simulink library "Renault_Library"  (Simulink 8.2)
settings: Solver=ode3  StartTime=0.0  StopTime=10.0  FixedStep=0.020  RelTol=1e-3
undefined symbols (not defined in the file or in its folder): ABC, AssystemProposal, b, chkval, CoeffA_C, CoeffB_C, CoeffMean_C, ConfTime_C, DiagDelay_C, DividePercent, FailureConfActive_C, FallingEdgeDuration_C, HighLevelThresholdVoltage_C, HighLevelVoltage_C, HighLevelVoltageTunable_C, InitValue_C, InputVoltRangeMax_C, InputVoltRangeMin_C, LowActiveContact_C, LowLevelThresholdVoltage_C, LowLevelVoltage_C, MaxOperatingVoltage_C, MinOperatingVoltage_C, mV2V_conversion, OUT1, OutOfRangeHigh_C, OutOfRangeLow_C, PinSupplySaturationMax, PinSupplySaturationMin, Res1Value_C, Res2Value_C, Res3Value_C, RisingEdgeDuration_C, RSA_CANSignalFailMonitoring, SampleTime_C, service14Table, service19Table, service22Table, service24Table, service28Table, service2ETable, service2FTable, service31Table, service87Table, SizeXStateDID2FTable, SizeXTable14, SizeXTable19, SizeXTable22, SizeXTable24, SizeXTable28, SizeXTable2E, SizeXTable2F, SizeXTable31, SizeXTable87, SizeYStateDID2FTable, SizeYTable14, SizeYTable19, SizeYTable22, SizeYTable24, SizeYTable28 ... (+22)
undefined symbols (not defined in the file): BatteryVoltage_ActivationThreshold_C, FrameStatus_AbsentValue_C, FrameStatus_ConfAbsentValue_C, MemThreshold_C, NAMEOFTHEMODEL_SAMPLE_TIME_CST, NbAbs1_C, NbConfAbs1_C, NbConfPres1_C, NbPres1_C, NOMDUSIGNAL_FaultConfTime_C, NOMDUSIGNAL_FaultRehabTime_C, NOMDUSIGNAL_switchvalue_C, NOMDUSIGNAL_unavailablevalue_C, PeriodFrame1_C, ResetTime_C, WakeUpTime_C
libraries: Renault_Library x112, simulink x75
(part 14 of 32)

== system Renault_Library/Services ECU/Mux/RSA_ConvertToMux ==
  DATA : Inport
      settings: AttributesFormatString=Type : %<OutDataTypeStr>
  Enable : EnablePort  StatesWhenEnabling=reset
  Compare : RelationalOperator  Operator===  OutDataTypeStr=boolean  InputSameDT=on
  Constant : Constant  DisableCoverage=on  Value=0  OutDataTypeStr=Inherit: Inherit via back propagation  SampleTime=-1  PreserveConstantTs=on
  Constant1 : Constant  Value=Offset  OutDataTypeStr=Inherit: Inherit via back propagation  SampleTime=-1
  Constant2 : Constant  Value=Divisor  OutDataTypeStr=Inherit: Inherit via back propagation  SampleTime=-1
  Constant3 : Constant  Value=Factor  OutDataTypeStr=Inherit: Inherit via back propagation  SampleTime=-1
  Constant4 : Constant  OutDataTypeStr=Inherit: Inherit via back propagation  SampleTime=-1
  Divide : Product  Inputs=*/  OutDataTypeStr=Inherit: Inherit via back propagation  RndMeth=Zero  InputSameDT=on
      settings: AttributesFormatString=Saturation:%<SaturateOnIntegerOverflow>
  Product : Product  OutDataTypeStr=Inherit: Inherit via back propagation  RndMeth=Zero  InputSameDT=on
      settings: AttributesFormatString=Saturation:%<SaturateOnIntegerOverflow>
  Sum : Sum  Inputs=+-  OutDataTypeStr=Inherit: Inherit via back propagation  RndMeth=Zero  InputSameDT=on
      settings: AttributesFormatString=Saturation:%<SaturateOnIntegerOverflow>
  Switch : Switch  Criteria=u2 ~= 0  RndMeth=Zero  InputSameDT=on
      settings: AttributesFormatString=Threshold : %<Threshold>\nSaturation:%<SaturateOnIntegerOverflow>
  SIGNAL : Outport  OutputWhenDisabled=reset  InitialOutput=0
      settings: AttributesFormatString=Type : %<OutDataTypeStr>\nInit :%<InitialOutput>
  wires:
  Constant/1 -> Compare/2
  Switch/1 -> Divide/2
  Constant4/1 -> Switch/1
  Compare/1 -> Switch/2
  Divide/1 -> SIGNAL/1
  Constant2/1 -> Compare/1, Switch/3
  Sum/1 -> Divide/1
  Constant1/1 -> Sum/2
  Product/1 -> Sum/1
  Constant3/1 -> Product/2
  DATA/1 -> Product/1

== system Renault_Library/Services ECU/Mux/RSA_FrameMonitoring ==
  FRAME_RECEIVED : Inport
      settings: AttributesFormatString=Type : %<OutDataTypeStr>
  Constant : Constant  Value=INCREMENT_COUNTER  OutDataTypeStr=Inherit: Inherit via back propagation  SampleTime=-1
  Constant1 : Constant  Value=MAX_COUNTER  OutDataTypeStr=Inherit: Inherit via back propagation  SampleTime=-1
  Constant2 : Constant  Value=MIN_COUNTER  OutDataTypeStr=Inherit: Inherit via back propagation  SampleTime=-1
  RSA_DetectChange : Renault_Library/Complex blocks/Logic & Bit Operations/RSA_DetectChange  Tag=[LIB]  vinit=0
  RelationalOperator : RelationalOperator  Operator===  OutDataTypeStr=boolean  InputSameDT=on
  Sum : Sum  OutDataTypeStr=Inherit: Inherit via back propagation  RndMeth=Zero  InputSameDT=on
      settings: AttributesFormatString=Saturation:%<SaturateOnIntegerOverflow>
  Switch : Switch  Criteria=u2 ~= 0  RndMeth=Zero  InputSameDT=on
      settings: AttributesFormatString=Threshold : %<Threshold>\nSaturation:%<SaturateOnIntegerOverflow>\nCriteria:%<Criteria>
  Switch1 : Switch  Criteria=u2 ~= 0  RndMeth=Zero  InputSameDT=on
      settings: AttributesFormatString=Threshold : %<Threshold>\nSaturation:%<SaturateOnIntegerOverflow>\nCriteria:%<Criteria>
  UnitDelay : UnitDelay  SampleTime=-1
      settings: AttributesFormatString=Initial value : %<X0>
  UnitDelay1 : UnitDelay  SampleTime=-1
      settings: AttributesFormatString=Initial value : %<X0>
  FRAME_SEND : Outport  OutputWhenDisabled=reset  InitialOutput=0
      settings: AttributesFormatString=Type : %<OutDataTypeStr>\nInit :%<InitialOutput>
  wires:
  Switch/1 -> FRAME_SEND/1, UnitDelay/1, UnitDelay1/1
  Constant/1 -> Sum/2
  RSA_DetectChange/1 -> Switch/2
  FRAME_RECEIVED/1 -> RSA_DetectChange/1
  UnitDelay/1 -> Switch/3
  UnitDelay1/1 -> Sum/1
  Sum/1 -> RelationalOperator/1, Switch1/3
  Constant1/1 -> RelationalOperator/2
  Constant2/1 -> Switch1/1
  RelationalOperator/1 -> Switch1/2
  Switch1/1 -> Switch/1

== system Renault_Library/Services ECU/Mux/RSA_FrameStatusMgt ==
  note: Frame_Update is equal to 1
      | when a frame has been received
      | (=1 each time the RX counter is incremented)
  note: Frame_Status:
      | = 1 for normally absent (absent but not expected) and init
      | = 2 for present
      | = 3 for anormally absent (absent and expected)
      | = 4 for anormally absent confirmed (absent and expected confirmed)
  note: A delay of NbAbs*Period*TimeOut is waited to declare an absent frame
      | A delay of NbConfAbs*Period*TimeOut is waited to confirm an absent frame
      | If NbConfPres = 0, there is no delay waited to rehabilite the frame
      | If NbPres = 0, stateflow is continuously waiting (without going back to ConfirmedAbsente State)
      | a NbConfPres Time to rehabilite the frame
      |
      | NbAbs, NbConf, Period and SampleTime are configurable parameters
  note: Fault_Management_Allowed = 1 when ECU is ON
      | Or when GADE is enabled
  Fault_Management_Allowed : Inport
  Frame_Update : Inport  Port=2
  DataTypeConversion : DataTypeConversion  RndMeth=Zero
      settings: AttributesFormatString=Saturation:%<SaturateOnIntegerOverflow>
  DataTypeConversion1 : DataTypeConversion  RndMeth=Zero
      settings: AttributesFormatString=Saturation:%<SaturateOnIntegerOverflow>
  FrameMonitoring : Stateflow chart  TreatAsAtomicUnit=on
  NbAbsCalib : Constant  Value=NbAbsCalib
  NbConfCalib : Constant  Value=NbConfCalib
  NbConfPresCalib : Constant  Value=NbConfPresCalib
  NbPresCalib : Constant  Value=NbPresCalib
  PeriodCalib : Constant  Value=PeriodCalib
  SampleTimeCalib : Constant  Value=SampleTimeCalib
  Terminator : Terminator
  Terminator1 : Terminator
  Frame_Status : Outport  InitialOutput=1
  wires:
  NbConfPresCalib/1 -> FrameMonitoring/8(NbConfPres)
  NbPresCalib/1 -> FrameMonitoring/7(NbPres)
  Fault_Management_Allowed/1 -> DataTypeConversion/1
  Frame_Update/1 -> DataTypeConversion1/1
  FrameMonitoring/1(Frame_Status) -> Frame_Status/1
  NbAbsCalib/1 -> FrameMonitoring/5(NbAbs)
  NbConfCalib/1 -> FrameMonitoring/6(NbConfAbs)
  PeriodCalib/1 -> FrameMonitoring/4(Period)
  FrameMonitoring/2(Cpt) -> Terminator/1
  FrameMonitoring/3(Cpt2) -> Terminator1/1
  SampleTimeCalib/1 -> FrameMonitoring/3(Ts)
  DataTypeConversion/1 -> FrameMonitoring/1(Fault_Management_Allowed)
  DataTypeConversion1/1 -> FrameMonitoring/2(Frame_Update)

== system Renault_Library/Services ECU/Mux/RSA_FrameUpdate ==
  note: Frame_Update is equal to 1
      | when a frame has been received
      | (=1 each time the RX counter is incremented)
  Frame_RX : Inport
  Fault_Management_Allowed : Inport  Port=2
  FrameUpdateAlwaysRunning : Constant  Value=FrameUpdateAlwaysRunning  OutDataTypeStr=Inherit: Inherit via back propagation  SampleTime=-1
  LogicalOperator1 : Logic  OutDataTypeStr=boolean
  RSA_DetectChange1 : Renault_Library/Complex blocks/Logic & Bit Operations/RSA_DetectChange  Tag=[LIB]  vinit=0
  Switch : Switch  Criteria=u2 ~= 0  RndMeth=Zero  InputSameDT=on
      settings: AttributesFormatString=Threshold : %<Threshold>\nSaturation:%<SaturateOnIntegerOverflow>\nCriteria:%<Criteria>
  Frame_Update : Outport  InitialOutput=0
  wires:
  Frame_RX/1 -> RSA_DetectChange1/1
  RSA_DetectChange1/1 -> LogicalOperator1/1, Switch/1
  Fault_Management_Allowed/1 -> LogicalOperator1/2
  Switch/1 -> Frame_Update/1
  FrameUpdateAlwaysRunning/1 -> Switch/2
  LogicalOperator1/1 -> Switch/3

== system Renault_Library/Services ECU/Mux/RSA_MuxMonitoring ==
  FRAME_RECEIVED : Inport  OutDataTypeStr=uint32
      settings: AttributesFormatString=Type : %<OutDataTypeStr>
  Enable : EnablePort
  CanFramePresence : Stateflow chart  TreatAsAtomicUnit=on
  FRAME_STATUS : Outport  OutDataTypeStr=uint32  OutputWhenDisabled=reset  InitialOutput=0
      settings: AttributesFormatString=Type : %<OutDataTypeStr>\nInit :%<InitialOutput>
  FRAME_PRESENT : Outport  Port=2  OutDataTypeStr=boolean  InitialOutput=0
      settings: AttributesFormatString=Type : %<OutDataTypeStr>\nInit :%<InitialOutput>
  wires:
  CanFramePresence/2(FRAME_PRESENT) -> FRAME_PRESENT/1
  CanFramePresence/1(FRAME_STATUS) -> FRAME_STATUS/1
  FRAME_RECEIVED/1 -> CanFramePresence/1(FRAME_RECEIVED)

== system Renault_Library/Services ECU/Mux/RSA_MuxSendFrame ==
  EVENT_DATA : Inport  OutDataTypeStr=boolean
      settings: AttributesFormatString=Type : %<OutDataTypeStr>
  Enable : EnablePort  StatesWhenEnabling=reset
  RSA_Send_Frame_FCT : Stateflow chart  TreatAsAtomicUnit=on
  FRAME_SEND : Outport  OutDataTypeStr=uint32  OutputWhenDisabled=reset  InitialOutput=0
      settings: AttributesFormatString=Type : %<OutDataTypeStr>\nInit :%<InitialOutput>
  FRAME_PRESENT : Outport  Port=2  OutDataTypeStr=boolean  OutputWhenDisabled=reset  InitialOutput=false
      settings: AttributesFormatString=Type : %<OutDataTypeStr>\nInit :%<InitialOutput>
  wires:
  RSA_Send_Frame_FCT/2(FRAME_PRESENT) -> FRAME_PRESENT/1
  EVENT_DATA/1 -> RSA_Send_Frame_FCT/1(EVENT_DATA)
  RSA_Send_Frame_FCT/1(CNT_FRAME_UPDATED) -> FRAME_SEND/1

== system Renault_Library/Services ECU/PowerSupply/RSA_PowerSupplyMgt ==
  note: Apc = PowerSupplyON = ECU activation switch
  note: EcuStatus= 0  --> Ecu off
      | EcuStatus= 1 --> Ecu on
      | EcuStatus= 2 --> Ecu Waking Up (Init)
      | EcuStatus= 3 --> Ecu Shutting Down (Reset)
  note: Read during Init or first step Ecu On when Init = 0
      | Write during Reset or last step Ecu On when Reset = 0
  note: ActivateEcu = 1 when BatteryVoltage > BattVoltageThreshold AND Apc is ON
      | ActivateEcu = 0 when Ecu is Off (EcuStatus = 0)
  note: WARNING: The writng operation is corresponding to powerlatch saving phase.
      | This need to be adapted for other specific writing sequence
  note: VoltageSupplyCutThreshold is a parameter to allow memorization when voltage is above its value.
      | If the voltage immediatly go under this threshold, there is no writing phase so no memorization.
      |
      | BattVoltageThreshold is a parameter that allow the activation of ECU.
      | If there is no voltage input, put the threshold to low value.
  BatteryVoltage : Inport
  Apc : Inport  Port=2
  BattVoltageThreshold : Constant  Value=BattVoltageThresholdCalib
  PowerSupMgt_Chart : Stateflow chart  TreatAsAtomicUnit=on
  ResetTimeCalib : Constant  Value=ResetTimeCalib
  SampleTimeCalib : Constant  Value=SampleTimeCalib
  VoltageSupplyCutThresholdCalib : Constant  Value=VoltageSupplyCutThresholdCalib
  WakeUpTimeCalib : Constant  Value=WakeUpTimeCalib
  ActivateEcu : Outport
  EcuStatus : Outport  Port=2
  Read : Outport  Port=3
  Write : Outport  Port=4
  wires:
  BattVoltageThreshold/1 -> PowerSupMgt_Chart/3(BatteryVoltageThreshold)
  PowerSupMgt_Chart/1(ActivateEcu) -> ActivateEcu/1
  PowerSupMgt_Chart/4(write) -> Write/1
  Apc/1 -> PowerSupMgt_Chart/1(Apc)
  PowerSupMgt_Chart/3(read) -> Read/1
  BatteryVoltage/1 -> PowerSupMgt_Chart/2(BatteryVoltage)
  PowerSupMgt_Chart/2(EcuStatus) -> EcuStatus/1
  WakeUpTimeCalib/1 -> PowerSupMgt_Chart/5(WakeUpTime)
  ResetTimeCalib/1 -> PowerSupMgt_Chart/6(ResetTime)
  VoltageSupplyCutThresholdCalib/1 -> PowerSupMgt_Chart/4(VoltageSupplyCutThreshold)
  SampleTimeCalib/1 -> PowerSupMgt_Chart/7(SampleTime)

== system Renault_Library/Standard blocks/Commonly Used Blocks/Subsystem ==
  In1 : Inport
  Out1 : Outport
  wires:
  In1/1 -> Out1/1

== system Renault_Library/Standard blocks/Discontinuities/SaturationDynamic ==
  up : Inport
      settings: AttributesFormatString=Type : %<OutDataTypeStr>
  u : Inport  Port=2
      settings: AttributesFormatString=Type : %<OutDataTypeStr>
  lo : Inport  Port=3
      settings: AttributesFormatString=Type : %<OutDataTypeStr>
  LowerRelop1 : RelationalOperator  DisableCoverage=on  Operator=>  OutDataTypeStr=boolean  InputSameDT=on
  Switch : Switch  Criteria=u2 ~= 0  OutDataTypeStr=Inherit: Inherit via back propagation  RndMeth=Zero  ZeroCross=off  InputSameDT=on
  Switch2 : Switch  Criteria=u2 ~= 0  OutMin=OutMin  OutMax=OutMax  OutDataTypeStr=Inherit: Inherit via back propagation  RndMeth=Zero  ZeroCross=off  InputSameDT=on
  UpperRelop : RelationalOperator  DisableCoverage=on  Operator=<  OutDataTypeStr=boolean  InputSameDT=on
  y : Outport
      settings: AttributesFormatString=Type : %<OutDataTypeStr>\nInit :%<InitialOutput>
  wires:
  [up] -> Switch2/1
  [u] -> UpperRelop/1
  [u] -> Switch/3
  [lo] -> Switch/1
  up/1 -> [up], LowerRelop1/2
  u/1 -> LowerRelop1/1, [u]
  lo/1 -> [lo], UpperRelop/2
  Switch/1 -> Switch2/3
  Switch2/1 -> y/1
  LowerRelop1/1 -> Switch2/2
  UpperRelop/1 -> Switch/2

== system Renault_Library/Standard blocks/Logic & Bit Operations/Extract Bits ==
  u : Inport
  DTProp1 : simulink/Signal Attributes/Data Type Propagation  PropDataType=fixdt(1, 16)  IfRefDouble=double  IfRefSingle=single  IsSigned=IsSigned1  NumBitsBase=NumBits1  NumBitsMult=0.5  NumBitsAdd=0  NumBitsAllowFinal=1:128  PropScaling=2^-10  ValuesUsedBestPrec=[5 -7]  SlopeBase=PosRange1  SlopeMult=2^0  SlopeAdd=0  BiasBase=Bias1  BiasMult=1  BiasAdd=0
  DTProp2 : simulink/Signal Attributes/Data Type Propagation  PropDataType=fixdt(1, 16)  IfRefDouble=double  IfRefSingle=single  IsSigned=IsSigned1  NumBitsBase=NumBits1  NumBitsMult=1  NumBitsAdd=0  NumBitsAllowFinal=1:128  PropScaling=1  ValuesUsedBestPrec=[5 -7]  SlopeBase=Slope1  SlopeMult=1  SlopeAdd=0  BiasBase=Bias1  BiasMult=1  BiasAdd=0
  Extract Desired Bits : DataTypeConversion  DisableCoverage=on
  Modify Scaling Only : DataTypeConversion  ConvertRealWorld=Stored Integer (SI)
  y : Outport
  wires:
  Modify Scaling Only/1 -> DTProp2/3, y/1
  u/1 -> Extract Desired Bits/1, DTProp1/2, DTProp1/1
  Extract Desired Bits/1 -> DTProp2/1, DTProp2/2, Modify Scaling Only/1, DTProp1/3

== system Renault_Library/Standard blocks/Model Wide Utilities/DocBlock ==
  EmptySubsystem : Subsystem

== system Renault_Library/Standard blocks/Model Wide Utilities/ModelInfo ==
  EmptySubsystem : Subsystem

== system Renault_Library/Standard blocks/Ports & Subsystems/AtomicSubsystem ==
  In : Inport
  Out : Outport
  wires:
  In/1 -> Out/1

== system Renault_Library/Standard blocks/Ports & Subsystems/CodeReuseSubsystem ==
  In1 : Inport
  Out1 : Outport
  wires:
  In1/1 -> Out1/1

== system Renault_Library/Standard blocks/Ports & Subsystems/EnabledSubsystem ==
  In1 : Inport
  Enable : EnablePort
      settings: AttributesFormatString=State : %<StatesWhenEnabling>
  Out1 : Outport
  wires:
  In1/1 -> Out1/1

== system Renault_Library/Standard blocks/Ports & Subsystems/EnabledandTriggeredSubsystem ==
  In1 : Inport
  Enable : EnablePort
  Trigger : TriggerPort
  Out1 : Outport
  wires:
  In1/1 -> Out1/1

== system Renault_Library/Standard blocks/Ports & Subsystems/ForIteratorSubsystem ==
  In1 : Inport
  ForIterator : ForIterator
  Out1 : Outport
  wires:
  In1/1 -> Out1/1

== system Renault_Library/Standard blocks/Ports & Subsystems/FunctionCallSubsystem ==
  In1 : Inport
  function_call : TriggerPort  TriggerType=function-call
  Out1 : Outport
  wires:
  In1/1 -> Out1/1

== system Renault_Library/Standard blocks/Ports & Subsystems/IfActionSubsystem ==
  In1 : Inport
  ActionPort : ActionPort
      settings: AttributesFormatString=State : %<InitializeStates>
  Out1 : Outport
  wires:
  In1/1 -> Out1/1

== system Renault_Library/Standard blocks/Ports & Subsystems/Subsystem ==
  In1 : Inport
  Out1 : Outport
  wires:
  In1/1 -> Out1/1

== system Renault_Library/Standard blocks/Ports & Subsystems/SwitchCaseActionSubsystem ==
  In1 : Inport
  ActionPort : ActionPort
  Out1 : Outport
  wires:
  In1/1 -> Out1/1

== system Renault_Library/Standard blocks/Ports & Subsystems/SwitchCaseActionSubsystem1 ==
  In1 : Inport
  ActionPort : ActionPort
  Out1 : Outport
  wires:
  In1/1 -> Out1/1

== system Renault_Library/Standard blocks/Ports & Subsystems/TriggeredSubsystem ==
  In1 : Inport
  Trigger : TriggerPort
      settings: AttributesFormatString=State : %<StatesWhenEnabling>
  Out1 : Outport
  wires:
  In1/1 -> Out1/1

== system Renault_Library/Standard blocks/Ports & Subsystems/Variant_Subsystem ==
  note: 1) Only subsystems can be added as variant choices at this level
      | 2) Blocks cannot be connected at this level as connectivity is
      | automatically determined at simulation, based on the active variant
  In : Inport
  Out : Outport

== system Renault_Library/Standard blocks/Ports & Subsystems/WhileIteratorSubsystem ==
  IC : Inport
  In1 : Inport  Port=2
  WhileIterator : WhileIterator
  Out1 : Outport
  wires:
  In1/1 -> Out1/1
  IC/1 -> WhileIterator/2

== system Renault_Library/Complex blocks/Discontinuities/RSA_Hysteresis_WithConf_V01_00/TurnOnDelay1 ==
  Delaytime : Inport  Tag=AM,In,0,1,Name,C;CMP,Port,PortWidth,SampleTime;
      settings: AttributesFormatString=Type : %<OutDataTypeStr>
  Signal : Inport  Tag=AM,In,0,1,Name,C;CMP,Port,PortWidth,SampleTime;  Port=2
      settings: AttributesFormatString=Type : %<OutDataTypeStr>
  Timestep1 : Inport  Tag=AM,In,0,1,Name,C;CMP,Port,PortWidth,SampleTime;  Port=3
      settings: AttributesFormatString=Type : %<OutDataTypeStr>
  Constant1 : Constant  Tag=AM,Constant,0,1,Value;CMP,Value;  Value=TIM1INI  OutDataTypeStr=Inherit: Inherit via back propagation
  Constant2 : Constant  Value=CONS_ONE  OutDataTypeStr=Inherit: Inherit via back propagation
  Constant3 : Constant  Value=TIM1INI  OutDataTypeStr=Inherit: Inherit via back propagation
  Constant4 : Constant  Value=CONS_ONE  OutDataTypeStr=Inherit: Inherit via back propagation
  Constant6 : Constant  Value=CONS_ZERO  OutDataTypeStr=Inherit: Inherit via back propagation
  Constant7 : Constant  Value=BOOSTING_FLP  OutDataTypeStr=uint32
  Constant8 : Constant  Value=BOOSTING_FXP  OutDataTypeStr=Inherit: Inherit via back propagation
  DataTypeConversion2 : DataTypeConversion  RndMeth=Zero
  DelayInput1 : UnitDelay  Description=\nStore in Global RAM  InitialCondition=timeoutinit  SampleTime=-1
      settings: AttributesFormatString=IC = %<X0>
  DelayInput2 : UnitDelay  Description=\nStore in Global RAM  InitialCondition=TIM1INI  SampleTime=-1
      settings: AttributesFormatString=IC = %<X0>
  EdgeRising : Subsystem  mask(EdgeRising){vinit=initinit}
      mask prompts: vinit "Initialisation"
      mask help: When a rising edge is used as input, the ouput is equal to one during the fall of the signal. Otherwise the output is zero.
      mask Display:
      | plot([0.2 0.4 0.4 0.6],[0.6 0.6 0.9 0.9]);
      | plot([0.2 0.4 0.4 0.4 0.6],[0.1 0.1 0.4 0.1 0.1])
  LogicalOperator : Logic  Tag=AM,Std,Inputs,1;CMP,Operator,Inputs;  OutDataTypeStr=boolean
  LogicalOperator1 : Logic  OutDataTypeStr=boolean
  Mux : Mux  Inputs=2
  Product : Product  OutDataTypeStr=Inherit: Inherit via back propagation  SaturateOnIntegerOverflow=on  RndMeth=Zero
  Product1 : Product  OutDataTypeStr=Inherit: Inherit via back propagation  SaturateOnIntegerOverflow=on  RndMeth=Zero
  Product2 : Product  OutDataTypeStr=uint32  SaturateOnIntegerOverflow=on  RndMeth=Zero
  RelationalOperator1 : RelationalOperator  Operator=>  OutDataTypeStr=boolean  ZeroCross=off  InputSameDT=on
  RelationalOperator2 : RelationalOperator  Operator=<=  OutDataTypeStr=boolean  ZeroCross=off  InputSameDT=on
  RelationalOperator3 : RelationalOperator  Operator=>  OutDataTypeStr=boolean  ZeroCross=off  InputSameDT=on
  Subtract4 : Sum  Inputs=-+  OutDataTypeStr=Inherit: Inherit via back propagation  RndMeth=Zero  InputSameDT=on
      settings: AttributesFormatString=SAT = %<SaturateOnIntegerOverflow>
  Sum : Sum  Inputs=+-  OutDataTypeStr=Inherit: Inherit via back propagation  RndMeth=Zero  InputSameDT=on
      settings: AttributesFormatString=SAT = %<SaturateOnIntegerOverflow>
  Switch{2..6} (5 alike) : Switch  Tag=GEN,Threshold;AM,Switch,2,1;CMP,Threshold;  Criteria=u2 ~= 0  RndMeth=Zero  SaturateOnIntegerOverflow=on  InputSameDT=on
      settings: AttributesFormatString=Criteria=%<Criteria>
  Terminator : Terminator
  Terminator1 : Terminator
  Terminator2 : Terminator
  Out : Outport  Tag=AM,Out,1,0,Name,NC;CMP,Port,OutputWhenDisabled,InitialOutput;
      settings: AttributesFormatString=Type : %<OutDataTypeStr>\nInit :%<InitialOutput>
  wires:
  Product/1 -> [Timestep1]
  Constant1/1 -> Switch5/3
  Switch5/1 -> Switch3/3
  RelationalOperator2/1 -> Switch5/2
  [EdgeRising] -> Switch3/2
  LogicalOperator1/1 -> LogicalOperator/2  "Timeout"
  Timestep1/1 -> Mux/1, Product/1, [Timestep]
  DelayInput1/1 -> Switch2/3
  Switch4/1 -> DelayInput2/1
  RelationalOperator1/1 -> Switch4/2, LogicalOperator1/2
  DelayInput2/1 -> RelationalOperator2/2, Sum/1
  Switch2/1 -> LogicalOperator1/1, DelayInput1/1
  Switch3/1 -> RelationalOperator1/2, Switch4/3
  EdgeRising/1(Out) -> [EdgeRising], Switch2/1, Switch2/2
  Signal/1 -> EdgeRising/1(In), LogicalOperator/1
  [Timestep1] -> Sum/2, RelationalOperator2/1
  Constant3/1 -> Switch4/1
  LogicalOperator/1 -> Out/1
  Mux/1 -> Terminator/1
  [Timestep] -> Product2/2, Subtract4/2
  Subtract4/1 -> RelationalOperator3/1
  [Timestep1] -> RelationalOperator1/1
  Constant6/1 -> RelationalOperator3/2
  Constant8/1 -> Switch6/3
  Constant7/1 -> Switch6/1
  Sum/1 -> Switch5/1
  Switch6/1 -> [tolcons]
  DataTypeConversion2/1 -> Subtract4/1
  Product2/1 -> DataTypeConversion2/1
  [CONS_ONE] -> Product1/2
  RelationalOperator3/1 -> Switch6/2
  Product1/1 -> Switch3/1
  Delaytime/1 -> Mux/2, Product1/1
  [CONS_ONE] -> Product/2
  Constant2/1 -> Product2/1
  [tolcons] -> Terminator1/1
  [CONS_ONE] -> Terminator2/1
  Constant4/1 -> [CONS_ONE]

== system Renault_Library/Complex blocks/Discontinuities/RSA_Hysteresis_WithConf_V01_00/TurnOnDelay2 ==
  Delaytime : Inport  Tag=AM,In,0,1,Name,C;CMP,Port,PortWidth,SampleTime;
      settings: AttributesFormatString=Type : %<OutDataTypeStr>
  Signal : Inport  Tag=AM,In,0,1,Name,C;CMP,Port,PortWidth,SampleTime;  Port=2
      settings: AttributesFormatString=Type : %<OutDataTypeStr>
  Timestep1 : Inport  Tag=AM,In,0,1,Name,C;CMP,Port,PortWidth,SampleTime;  Port=3
      settings: AttributesFormatString=Type : %<OutDataTypeStr>
  Constant1 : Constant  Tag=AM,Constant,0,1,Value;CMP,Value;  Value=TIM1INI  OutDataTypeStr=Inherit: Inherit via back propagation
  Constant2 : Constant  Value=CONS_ONE  OutDataTypeStr=Inherit: Inherit via back propagation
  Constant3 : Constant  Value=TIM1INI  OutDataTypeStr=Inherit: Inherit via back propagation
  Constant4 : Constant  Value=CONS_ONE  OutDataTypeStr=Inherit: Inherit via back propagation
  Constant6 : Constant  Value=CONS_ZERO  OutDataTypeStr=Inherit: Inherit via back propagation
  Constant7 : Constant  Value=BOOSTING_FLP  OutDataTypeStr=uint32
  Constant8 : Constant  Value=BOOSTING_FXP  OutDataTypeStr=Inherit: Inherit via back propagation
  DataTypeConversion2 : DataTypeConversion  RndMeth=Zero
  DelayInput1 : UnitDelay  Description=\nStore in Global RAM  InitialCondition=timeoutinit  SampleTime=-1
      settings: AttributesFormatString=IC = %<X0>
  DelayInput2 : UnitDelay  Description=\nStore in Global RAM  InitialCondition=TIM1INI  SampleTime=-1
      settings: AttributesFormatString=IC = %<X0>
  EdgeRising : Subsystem  mask(EdgeRising){vinit=initinit}
      mask prompts: vinit "Initialisation"
      mask help: When a rising edge is used as input, the ouput is equal to one during the fall of the signal. Otherwise the output is zero.
      mask Display:
      | plot([0.2 0.4 0.4 0.6],[0.6 0.6 0.9 0.9]);
      | plot([0.2 0.4 0.4 0.4 0.6],[0.1 0.1 0.4 0.1 0.1])
  LogicalOperator : Logic  Tag=AM,Std,Inputs,1;CMP,Operator,Inputs;  OutDataTypeStr=boolean
  LogicalOperator1 : Logic  OutDataTypeStr=boolean
  Mux : Mux  Inputs=2
  Product : Product  OutDataTypeStr=Inherit: Inherit via back propagation  SaturateOnIntegerOverflow=on  RndMeth=Zero
  Product1 : Product  OutDataTypeStr=Inherit: Inherit via back propagation  SaturateOnIntegerOverflow=on  RndMeth=Zero
  Product2 : Product  OutDataTypeStr=uint32  SaturateOnIntegerOverflow=on  RndMeth=Zero
  RelationalOperator1 : RelationalOperator  Operator=>  OutDataTypeStr=boolean  ZeroCross=off  InputSameDT=on
  RelationalOperator2 : RelationalOperator  Operator=<=  OutDataTypeStr=boolean  ZeroCross=off  InputSameDT=on
  RelationalOperator3 : RelationalOperator  Operator=>  OutDataTypeStr=boolean  ZeroCross=off  InputSameDT=on
  Subtract4 : Sum  Inputs=-+  OutDataTypeStr=Inherit: Inherit via back propagation  RndMeth=Zero  InputSameDT=on
      settings: AttributesFormatString=SAT = %<SaturateOnIntegerOverflow>
  Sum : Sum  Inputs=+-  OutDataTypeStr=Inherit: Inherit via back propagation  RndMeth=Zero  InputSameDT=on
      settings: AttributesFormatString=SAT = %<SaturateOnIntegerOverflow>
  Switch{2..6} (5 alike) : Switch  Tag=GEN,Threshold;AM,Switch,2,1;CMP,Threshold;  Criteria=u2 ~= 0  RndMeth=Zero  SaturateOnIntegerOverflow=on  InputSameDT=on
      settings: AttributesFormatString=Criteria=%<Criteria>
  Terminator : Terminator
  Terminator1 : Terminator
  Terminator2 : Terminator
  Out : Outport  Tag=AM,Out,1,0,Name,NC;CMP,Port,OutputWhenDisabled,InitialOutput;
      settings: AttributesFormatString=Type : %<OutDataTypeStr>\nInit :%<InitialOutput>
  wires:
  Product/1 -> [Timestep1]
  Constant1/1 -> Switch5/3
  Switch5/1 -> Switch3/3
  RelationalOperator2/1 -> Switch5/2
  [EdgeRising] -> Switch3/2
  LogicalOperator1/1 -> LogicalOperator/2  "Timeout"
  Timestep1/1 -> Mux/1, Product/1, [Timestep]
  DelayInput1/1 -> Switch2/3
  Switch4/1 -> DelayInput2/1
  RelationalOperator1/1 -> Switch4/2, LogicalOperator1/2
  DelayInput2/1 -> RelationalOperator2/2, Sum/1
  Switch2/1 -> LogicalOperator1/1, DelayInput1/1
  Switch3/1 -> RelationalOperator1/2, Switch4/3
  EdgeRising/1(Out) -> [EdgeRising], Switch2/1, Switch2/2
  Signal/1 -> EdgeRising/1(In), LogicalOperator/1
  [Timestep1] -> Sum/2, RelationalOperator2/1
  Constant3/1 -> Switch4/1
  LogicalOperator/1 -> Out/1
  Mux/1 -> Terminator/1
  [Timestep] -> Product2/2, Subtract4/2
  Subtract4/1 -> RelationalOperator3/1
  [Timestep1] -> RelationalOperator1/1
  Constant6/1 -> RelationalOperator3/2
  Constant8/1 -> Switch6/3
  Constant7/1 -> Switch6/1
  Sum/1 -> Switch5/1
  Switch6/1 -> [tolcons]
  DataTypeConversion2/1 -> Subtract4/1
  Product2/1 -> DataTypeConversion2/1
  [CONS_ONE] -> Product1/2
  RelationalOperator3/1 -> Switch6/2
  Product1/1 -> Switch3/1
  Delaytime/1 -> Mux/2, Product1/1
  [CONS_ONE] -> Product/2
  Constant2/1 -> Product2/1
  [tolcons] -> Terminator1/1
  [CONS_ONE] -> Terminator2/1
  Constant4/1 -> [CONS_ONE]

== system Renault_Library/Complex blocks/Lookup Tables/RSA_LookupTableDynamic_v02_01/Sat_blk ==
  Threshold_Upper : Inport
      settings: AttributesFormatString=Type : %<OutDataTypeStr>
  In : Inport  Port=2
      settings: AttributesFormatString=Type : %<OutDataTypeStr>
  Min : MinMax  Inputs=2  OutDataTypeStr=Inherit: Inherit via back propagation  RndMeth=Zero  SaturateOnIntegerOverflow=on  InputSameDT=on
  Out : Outport
      settings: AttributesFormatString=Type : %<OutDataTypeStr>\nInit :%<InitialOutput>
  wires:
  Threshold_Upper/1 -> [Threshold_Upper]
  In/1 -> Min/1
  Min/1 -> Out/1
  [Threshold_Upper] -> Min/2

== system Renault_Library/Complex blocks/Math Operations/RSA_DivisionByZero/Perform_Division ==
  num : Inport  Tag=AM,In,0,1,Name,C;CMP,Port,PortWidth,SampleTime;
      settings: AttributesFormatString=Type : %<OutDataTypeStr>
  den : Inport  Tag=AM,In,0,1,Name,C;CMP,Port,PortWidth,SampleTime;  Port=2
      settings: AttributesFormatString=Type : %<OutDataTypeStr>
  Enable_Division : EnablePort  Tag=GEN,StatesWhenEnabling;AM,In,0,1,Name,NC;CMP,StatesWhenEnabling;
      settings: AttributesFormatString=<states=%<statesWhenEnabling>>
  Divide : Product  Tag=[LIB]  Inputs=*/  OutDataTypeStr=Inherit: Inherit via back propagation  SaturateOnIntegerOverflow=on  RndMeth=Zero
      settings: AttributesFormatString=SAT = %<SaturateOnIntegerOverflow>
  ans : Outport  Tag=AM,Out,1,0,Name,NC;CMP,Port,OutputWhenDisabled,InitialOutput;  InitialOutput=0
      settings: AttributesFormatString=Init :%<InitialOutput>\nDisabled : %<OutputWhenDisabled>
  wires:
  Divide/1 -> ans/1
  den/1 -> Divide/2
  num/1 -> Divide/1

== system Renault_Library/Complex blocks/Signal Routing/RSA_SlopeSwitch2Inputs/Signal_Change_Compute ==
  Switch_Cmd : Inport
      settings: AttributesFormatString=Type : %<OutDataTypeStr>
  Relational_Operator : RelationalOperator  Operator=~=  OutDataTypeStr=boolean  InputSameDT=on
  UnitDelay : UnitDelay  InitialCondition=SS2ININI  SampleTime=-1
  Signal_Change : Outport
      settings: AttributesFormatString=Type : %<OutDataTypeStr>\nInit :%<InitialOutput>
  wires:
  Relational_Operator/1 -> Signal_Change/1
  Switch_Cmd/1 -> Relational_Operator/1, UnitDelay/1
  UnitDelay/1 -> Relational_Operator/2

== system Renault_Library/Complex blocks/Signal Routing/RSA_SlopeSwitch2Inputs/Slope_On_Block ==
  Signal_Change : Inport  Tag=AM,In,0,1,Name,C;CMP,Port,PortWidth,SampleTime;
      settings: AttributesFormatString=Type : %<OutDataTypeStr>
  Input : Inport  Tag=AM,In,0,1,Name,C;CMP,Port,PortWidth,SampleTime;  Port=2
      settings: AttributesFormatString=Type : %<OutDataTypeStr>
  Pos_Slope : Inport  Tag=AM,In,0,1,Name,C;CMP,Port,PortWidth,SampleTime;  Port=3
      settings: AttributesFormatString=Type : %<OutDataTypeStr>
  Neg_Slope : Inport  Tag=AM,In,0,1,Name,C;CMP,Port,PortWidth,SampleTime;  Port=4
      settings: AttributesFormatString=Type : %<OutDataTypeStr>
  Prev_Output : Inport  Port=5
      settings: AttributesFormatString=Type : %<OutDataTypeStr>
  Enable_Slope_On_Block : EnablePort  StatesWhenEnabling=reset
  Error_Due_To_High_Slope_Test : Subsystem
  Output_Comparision : Subsystem
  Output_Not_Equals_Input : Outport  Tag=AM,Out,1,0,Name,NC;CMP,Port,OutputWhenDisabled,InitialOutput;
      settings: AttributesFormatString=Init :%<InitialOutput>\nDisabled : %<OutputWhenDisabled>
  Output : Outport  Tag=AM,Out,1,0,Name,NC;CMP,Port,OutputWhenDisabled,InitialOutput;  Port=2
      settings: AttributesFormatString=Init :%<InitialOutput>\nDisabled : %<OutputWhenDisabled>
  wires:
  Output_Comparision/4(RelOp1_out) -> [RelOp1_out]
  Output_Comparision/3(RelOp2_out) -> [RelOp2_out]
  Neg_Slope/1 -> Output_Comparision/5(Neg_Slope)
  Input/1 -> Output_Comparision/2(Input)
  Pos_Slope/1 -> Output_Comparision/3(Pos_Slope)
  Prev_Output/1 -> Output_Comparision/4(Prev_Output)
  Error_Due_To_High_Slope_Test/1(Test_passed) -> Output_Comparision/1(Test_passed)
  Output_Comparision/2(Output) -> Output/1
  Output_Comparision/1(Output_Not_Equals_Input) -> Output_Not_Equals_Input/1
  [RelOp2_out] -> Error_Due_To_High_Slope_Test/3(RelOp2_out)
  [RelOp1_out] -> Error_Due_To_High_Slope_Test/2(RelOp1_out)
  Signal_Change/1 -> Error_Due_To_High_Slope_Test/1(Signal_Change)

== system Renault_Library/Complex blocks/Signal Routing/RSA_SlopeSwitch2Inputs/Slope_On_Compute ==
  Signal_Change : Inport  Tag=AM,In,0,1,Name,C;CMP,Port,PortWidth,SampleTime;
      settings: AttributesFormatString=Type : %<OutDataTypeStr>
  Output_Not_Equals_Input : Inport  Tag=AM,In,0,1,Name,C;CMP,Port,PortWidth,SampleTime;  Port=2
      settings: AttributesFormatString=Type : %<OutDataTypeStr>
  Constant1 : Constant  Value=false  OutDataTypeStr=Inherit: Inherit via back propagation  SampleTime=-1
  LogicalOperator : Logic  Operator=OR  OutDataTypeStr=boolean
  Switch1 : Switch  Tag=GEN,Threshold;AM,Switch,2,1;CMP,Threshold;  Criteria=u2 ~= 0  RndMeth=Zero  SaturateOnIntegerOverflow=on  InputSameDT=on
      settings: AttributesFormatString=Criteria=%<Criteria>
  UnitDelay3 : UnitDelay  InitialCondition=true  SampleTime=-1
  Slope_On : Outport  Tag=AM,Out,1,0,Name,NC;CMP,Port,OutputWhenDisabled,InitialOutput;
      settings: AttributesFormatString=Type : %<OutDataTypeStr>\nInit :%<InitialOutput>
  wires:
  Signal_Change/1 -> Switch1/3
  Constant1/1 -> UnitDelay3/1, Switch1/1
  Switch1/1 -> LogicalOperator/2
  UnitDelay3/1 -> Switch1/2
  LogicalOperator/1 -> Slope_On/1
  Output_Not_Equals_Input/1 -> LogicalOperator/1

== system Renault_Library/Complex blocks/Timers & Counters/RSA_Timer/EdgeRising ==
  In : Inport  Tag=AM,In,0,1,Name,C;CMP,Port,PortWidth,SampleTime;
      settings: AttributesFormatString=Type : %<OutDataTypeStr>
  DelayInput1 : UnitDelay  Description=\nStore in Global RAM  InitialCondition=vinit  SampleTime=-1
  LogicalOperator1 : Logic  OutDataTypeStr=boolean
  LogicalOperator3 : Logic  Operator=NOT  Inputs=1  OutDataTypeStr=boolean
  Out : Outport  Tag=AM,Out,1,0,Name,NC;CMP,Port,OutputWhenDisabled,InitialOutput;
      settings: AttributesFormatString=Type : %<OutDataTypeStr>\nInit :%<InitialOutput>
  wires:
  LogicalOperator1/1 -> Out/1
  In/1 -> LogicalOperator1/1, DelayInput1/1
  DelayInput1/1 -> LogicalOperator3/1
  LogicalOperator3/1 -> LogicalOperator1/2

== system Renault_Library/Complex blocks/Timers & Counters/RSA_Timer1/EdgeRising ==
  In : Inport  Tag=AM,In,0,1,Name,C;CMP,Port,PortWidth,SampleTime;
      settings: AttributesFormatString=Type : %<OutDataTypeStr>
  DelayInput1 : UnitDelay  Description=\nStore in Global RAM  InitialCondition=vinit  SampleTime=-1
  LogicalOperator1 : Logic  OutDataTypeStr=boolean
  LogicalOperator3 : Logic  Operator=NOT  Inputs=1  OutDataTypeStr=boolean
  Out : Outport  Tag=AM,Out,1,0,Name,NC;CMP,Port,OutputWhenDisabled,InitialOutput;
      settings: AttributesFormatString=Type : %<OutDataTypeStr>\nInit :%<InitialOutput>
  wires:
  LogicalOperator3/1 -> LogicalOperator1/2
  DelayInput1/1 -> LogicalOperator3/1
  In/1 -> DelayInput1/1, LogicalOperator1/1
  LogicalOperator1/1 -> Out/1

== system Renault_Library/Complex blocks/Timers & Counters/RSA_Timer1_v01_04/EdgeRising ==
  In : Inport  Tag=AM,In,0,1,Name,C;CMP,Port,PortWidth,SampleTime;
      settings: AttributesFormatString=Type : %<OutDataTypeStr>
  DelayInput1 : UnitDelay  Description=\nStore in Global RAM  InitialCondition=vinit  SampleTime=-1
  LogicalOperator1 : Logic  OutDataTypeStr=boolean
  LogicalOperator3 : Logic  Operator=NOT  Inputs=1  OutDataTypeStr=boolean
  Out : Outport  Tag=AM,Out,1,0,Name,NC;CMP,Port,OutputWhenDisabled,InitialOutput;
      settings: AttributesFormatString=Type : %<OutDataTypeStr>\nInit :%<InitialOutput>
  wires:
  LogicalOperator3/1 -> LogicalOperator1/2
  DelayInput1/1 -> LogicalOperator3/1
  In/1 -> DelayInput1/1, LogicalOperator1/1
  LogicalOperator1/1 -> Out/1

== system Renault_Library/Complex blocks/Timers & Counters/RSA_Timer1_v01_05/EdgeRising ==
  In : Inport  Tag=AM,In,0,1,Name,C;CMP,Port,PortWidth,SampleTime;
      settings: AttributesFormatString=Type : %<OutDataTypeStr>
  DelayInput1 : UnitDelay  Description=\nStore in Global RAM  InitialCondition=vinit  SampleTime=-1
      settings: AttributesFormatString=IC = %<X0>
  LogicalOperator1 : Logic  OutDataTypeStr=boolean
  LogicalOperator3 : Logic  Operator=NOT  Inputs=1  OutDataTypeStr=boolean
  Out : Outport  Tag=AM,Out,1,0,Name,NC;CMP,Port,OutputWhenDisabled,InitialOutput;
      settings: AttributesFormatString=Type : %<OutDataTypeStr>\nInit :%<InitialOutput>
  wires:
  LogicalOperator3/1 -> LogicalOperator1/2
  DelayInput1/1 -> LogicalOperator3/1
  In/1 -> DelayInput1/1, LogicalOperator1/1
  LogicalOperator1/1 -> Out/1

== system Renault_Library/Complex blocks/Timers & Counters/RSA_Timer1_v01_06/EdgeRising ==
  In : Inport  Tag=AM,In,0,1,Name,C;CMP,Port,PortWidth,SampleTime;
      settings: AttributesFormatString=Type : %<OutDataTypeStr>
  DelayInput1 : UnitDelay  Description=\nStore in Global RAM  InitialCondition=vinit  SampleTime=-1
      settings: AttributesFormatString=IC = %<X0>
  LogicalOperator1 : Logic  OutDataTypeStr=boolean
  LogicalOperator3 : Logic  Operator=NOT  Inputs=1  OutDataTypeStr=boolean
  Out : Outport  Tag=AM,Out,1,0,Name,NC;CMP,Port,OutputWhenDisabled,InitialOutput;
      settings: AttributesFormatString=Type : %<OutDataTypeStr>\nInit :%<InitialOutput>
  wires:
  LogicalOperator3/1 -> LogicalOperator1/2
  DelayInput1/1 -> LogicalOperator3/1
  In/1 -> DelayInput1/1, LogicalOperator1/1
  LogicalOperator1/1 -> Out/1

== system Renault_Library/Complex blocks/Timers & Counters/RSA_Timer_v01_04/EdgeRising ==
  In : Inport  Tag=AM,In,0,1,Name,C;CMP,Port,PortWidth,SampleTime;
      settings: AttributesFormatString=Type : %<OutDataTypeStr>
  DelayInput1 : UnitDelay  Description=\nStore in Global RAM  InitialCondition=vinit  SampleTime=-1
      settings: AttributesFormatString=IC = %<X0>
  LogicalOperator1 : Logic  OutDataTypeStr=boolean
  LogicalOperator3 : Logic  Operator=NOT  Inputs=1  OutDataTypeStr=boolean
  Out : Outport  Tag=AM,Out,1,0,Name,NC;CMP,Port,OutputWhenDisabled,InitialOutput;
      settings: AttributesFormatString=Type : %<OutDataTypeStr>\nInit :%<InitialOutput>
  wires:
  LogicalOperator3/1 -> LogicalOperator1/2
  DelayInput1/1 -> LogicalOperator3/1
  In/1 -> DelayInput1/1, LogicalOperator1/1
  LogicalOperator1/1 -> Out/1

== system Renault_Library/Complex blocks/Timers & Counters/RSA_Timer_v01_05/EdgeRising ==
  In : Inport  Tag=AM,In,0,1,Name,C;CMP,Port,PortWidth,SampleTime;
      settings: AttributesFormatString=Type : %<OutDataTypeStr>
  DelayInput1 : UnitDelay  Description=\nStore in Global RAM  InitialCondition=vinit  SampleTime=-1
      settings: AttributesFormatString=IC = %<X0>
  LogicalOperator1 : Logic  OutDataTypeStr=boolean
  LogicalOperator3 : Logic  Operator=NOT  Inputs=1  OutDataTypeStr=boolean
  Out : Outport  Tag=AM,Out,1,0,Name,NC;CMP,Port,OutputWhenDisabled,InitialOutput;
      settings: AttributesFormatString=Type : %<OutDataTypeStr>\nInit :%<InitialOutput>
  wires:
  LogicalOperator3/1 -> LogicalOperator1/2
  DelayInput1/1 -> LogicalOperator3/1
  In/1 -> DelayInput1/1, LogicalOperator1/1
  LogicalOperator1/1 -> Out/1

== system Renault_Library/Complex blocks/Timers & Counters/RSA_Timer_v01_05/RSA_CompareToZero ==
  u : Inport
      settings: AttributesFormatString=Type : %<OutDataTypeStr>
  Compare : RelationalOperator  Operator=~=  OutDataTypeStr=boolean  InputSameDT=on
  Constant : Constant  DisableCoverage=on  Value=cons_zero  OutDataTypeStr=Inherit: Inherit via back propagation  SampleTime=-1  PreserveConstantTs=on
  y : Outport
      settings: AttributesFormatString=Type : %<OutDataTypeStr>\nInit :%<InitialOutput>
  wires:
  Compare/1 -> y/1
  u/1 -> Compare/1
  Constant/1 -> Compare/2

== system Renault_Library/Complex blocks/Timers & Counters/RSA_Timer_v01_05/RSA_DataTypeDuplicate_v01_03 ==
  In1 : Inport
  In2 : Inport  Port=2
  Mux : Mux  Inputs=2
  Terminator : Terminator
  wires:
  Mux/1 -> Terminator/1
  In1/1 -> Mux/1
  In2/1 -> Mux/2

== system Renault_Library/Complex blocks/Timers & Counters/RSA_Timer_v01_06/EdgeRising ==
  In : Inport  Tag=AM,In,0,1,Name,C;CMP,Port,PortWidth,SampleTime;
      settings: AttributesFormatString=Type : %<OutDataTypeStr>
  DelayInput1 : UnitDelay  Description=\nStore in Global RAM  InitialCondition=vinit  SampleTime=-1
      settings: AttributesFormatString=IC = %<X0>
  LogicalOperator1 : Logic  OutDataTypeStr=boolean
  LogicalOperator3 : Logic  Operator=NOT  Inputs=1  OutDataTypeStr=boolean
  Out : Outport  Tag=AM,Out,1,0,Name,NC;CMP,Port,OutputWhenDisabled,InitialOutput;
      settings: AttributesFormatString=Type : %<OutDataTypeStr>\nInit :%<InitialOutput>
  wires:
  LogicalOperator3/1 -> LogicalOperator1/2
  DelayInput1/1 -> LogicalOperator3/1
  In/1 -> DelayInput1/1, LogicalOperator1/1
  LogicalOperator1/1 -> Out/1

== system Renault_Library/Complex blocks/Timers & Counters/RSA_Timer_v01_07/EdgeRising ==
  In : Inport  Tag=AM,In,0,1,Name,C;CMP,Port,PortWidth,SampleTime;
      settings: AttributesFormatString=Type : %<OutDataTypeStr>
  DelayInput1 : UnitDelay  Description=\nStore in Global RAM  InitialCondition=vinit  SampleTime=-1
      settings: AttributesFormatString=IC = %<X0>
  LogicalOperator1 : Logic  OutDataTypeStr=boolean
  LogicalOperator3 : Logic  Operator=NOT  Inputs=1  OutDataTypeStr=boolean
  Out : Outport  Tag=AM,Out,1,0,Name,NC;CMP,Port,OutputWhenDisabled,InitialOutput;
      settings: AttributesFormatString=Type : %<OutDataTypeStr>\nInit :%<InitialOutput>
  wires:
  LogicalOperator3/1 -> LogicalOperator1/2
  DelayInput1/1 -> LogicalOperator3/1
  In/1 -> DelayInput1/1, LogicalOperator1/1
  LogicalOperator1/1 -> Out/1

== system Renault_Library/Complex blocks/Timers & Counters/RSA_TurnOffDelay_v01_00/FallingEdge ==
  In : Inport  Tag=AM,In,0,1,Name,C;CMP,Port,PortWidth,SampleTime;
      settings: AttributesFormatString=Type : %<OutDataTypeStr>
  DelayInput1 : UnitDelay  Description=\nStore in Global RAM  InitialCondition=initinit  SampleTime=-1
      settings: AttributesFormatString=IC = %<X0>
  LogicalOperator1 : Logic  OutDataTypeStr=boolean
  LogicalOperator3 : Logic  Operator=NOT  Inputs=1  OutDataTypeStr=boolean
  Out : Outport  Tag=AM,Out,1,0,Name,NC;CMP,Port,OutputWhenDisabled,InitialOutput;
      settings: AttributesFormatString=Type : %<OutDataTypeStr>\nInit :%<InitialOutput>
  wires:
  DelayInput1/1 -> LogicalOperator1/2
  In/1 -> LogicalOperator3/1, DelayInput1/1
  LogicalOperator3/1 -> LogicalOperator1/1
  LogicalOperator1/1 -> Out/1

== system Renault_Library/Complex blocks/Timers & Counters/RSA_TurnOnDelay2_v01_04/RSA_CompareToZero ==
  u : Inport
      settings: AttributesFormatString=Type : %<OutDataTypeStr>
  Compare : RelationalOperator  Operator=~=  OutDataTypeStr=boolean  InputSameDT=on
  Constant : Constant  DisableCoverage=on  Value=cons_zero  OutDataTypeStr=Inherit: Inherit via back propagation  SampleTime=-1  PreserveConstantTs=on
  y : Outport
      settings: AttributesFormatString=Type : %<OutDataTypeStr>\nInit :%<InitialOutput>
  wires:
  Compare/1 -> y/1
  u/1 -> Compare/1
  Constant/1 -> Compare/2

== system Renault_Library/Complex blocks/Timers & Counters/RSA_TurnOnDelay2_v01_04/RSA_DataTypeDuplicate_v01_03 ==
  In1 : Inport
  In2 : Inport  Port=2
  Mux : Mux  Inputs=2
  Terminator : Terminator
  wires:
  Mux/1 -> Terminator/1
  In1/1 -> Mux/1
  In2/1 -> Mux/2

== system Renault_Library/Complex blocks/Timers & Counters/RSA_TurnOnDelay_v01_04/EdgeRising ==
  In : Inport  Tag=AM,In,0,1,Name,C;CMP,Port,PortWidth,SampleTime;
      settings: AttributesFormatString=Type : %<OutDataTypeStr>
  DelayInput1 : UnitDelay  Description=\nStore in Global RAM  InitialCondition=vinit  SampleTime=-1
  LogicalOperator1 : Logic  OutDataTypeStr=boolean
  LogicalOperator3 : Logic  Operator=NOT  Inputs=1  OutDataTypeStr=boolean
  Out : Outport  Tag=AM,Out,1,0,Name,NC;CMP,Port,OutputWhenDisabled,InitialOutput;
      settings: AttributesFormatString=Type : %<OutDataTypeStr>\nInit :%<InitialOutput>
  wires:
  LogicalOperator3/1 -> LogicalOperator1/2
  DelayInput1/1 -> LogicalOperator3/1
  In/1 -> DelayInput1/1, LogicalOperator1/1
  LogicalOperator1/1 -> Out/1

== system Renault_Library/Complex blocks/Timers & Counters/RSA_TurnOnDelay_v01_05/EdgeRising ==
  In : Inport  Tag=AM,In,0,1,Name,C;CMP,Port,PortWidth,SampleTime;
      settings: AttributesFormatString=Type : %<OutDataTypeStr>
  DelayInput1 : UnitDelay  Description=\nStore in Global RAM  InitialCondition=vinit  SampleTime=-1
      settings: AttributesFormatString=IC = %<X0>
  LogicalOperator1 : Logic  OutDataTypeStr=boolean
  LogicalOperator3 : Logic  Operator=NOT  Inputs=1  OutDataTypeStr=boolean
  Out : Outport  Tag=AM,Out,1,0,Name,NC;CMP,Port,OutputWhenDisabled,InitialOutput;
      settings: AttributesFormatString=Type : %<OutDataTypeStr>\nInit :%<InitialOutput>
  wires:
  LogicalOperator3/1 -> LogicalOperator1/2
  DelayInput1/1 -> LogicalOperator3/1
  In/1 -> DelayInput1/1, LogicalOperator1/1
  LogicalOperator1/1 -> Out/1

== system Renault_Library/Services ECU/Commonly_Used_Blocks/RSA_ServECU_EnaOutContact/ActiveStateAdaptation ==
  LogicalState : Inport
  DataTypeConversion2 : DataTypeConversion  RndMeth=Zero
  LogicalOperator2 : Logic  Operator=NOT  OutDataTypeStr=boolean
  RiseTime3 : Constant  Value=LowActiveContact_C  OutDataTypeStr=Inherit: Inherit via back propagation  SampleTime=-1
  Switch1 : Switch  Criteria=u2 ~= 0  RndMeth=Zero  InputSameDT=on
  AdaptedLogicalState : Outport  OutputWhenDisabled=reset  InitialOutput=0
  wires:
  Switch1/1 -> AdaptedLogicalState/1
  LogicalState/1 -> DataTypeConversion2/1
  LogicalOperator2/1 -> Switch1/1
  RiseTime3/1 -> Switch1/2
  DataTypeConversion2/1 -> Switch1/3, LogicalOperator2/1

== system Renault_Library/Services ECU/Commonly_Used_Blocks/RSA_ServECU_EnaOutContact/SwitchWithSlope ==
  Switch_Cmd : Inport
  RiseTime : Inport  Port=2
  FallTime : Inport  Port=3
  LowLevelVoltage : Inport  Port=4
  HighLevelVoltage : Inport  Port=5
  Enable : EnablePort  StatesWhenEnabling=reset
  Add1 : Sum  Inputs=-+  RndMeth=Zero  InputSameDT=on
  Add2 : Sum  Inputs=-+  RndMeth=Zero  InputSameDT=on
  RSA_ADivB1 : Renault_Library/Complex blocks/Math Operations/RSA_ADivB  Tag=[LIB]
  RSA_ADivB2 : Renault_Library/Complex blocks/Math Operations/RSA_ADivB  Tag=[LIB]
  RSA_SlopeSwitch2Inputs : Renault_Library/Complex blocks/Signal Routing/RSA_SlopeSwitch2Inputs  Tag=[LIB]  st=SampleTimeSec_C
  Out1 : Outport  OutputWhenDisabled=reset  InitialOutput=0
  Out2 : Outport  Port=2  OutputWhenDisabled=reset  InitialOutput=0
  wires:
  LowLevelVoltage/1 -> [LowLevelVoltage], RSA_SlopeSwitch2Inputs/4
  HighLevelVoltage/1 -> [HighLevelVoltage], RSA_SlopeSwitch2Inputs/5
  Switch_Cmd/1 -> RSA_SlopeSwitch2Inputs/1
  RSA_SlopeSwitch2Inputs/1 -> Out1/1
  RSA_SlopeSwitch2Inputs/2 -> Out2/1
  [HighLevelVoltage] -> Add2/1
  Add2/1 -> RSA_ADivB1/1
  [LowLevelVoltage] -> Add2/2
  Add1/1 -> RSA_ADivB2/1
  [HighLevelVoltage] -> Add1/2
  [LowLevelVoltage] -> Add1/1
  RiseTime/1 -> RSA_ADivB2/2
  RSA_ADivB2/1 -> RSA_SlopeSwitch2Inputs/2
  RSA_ADivB1/1 -> RSA_SlopeSwitch2Inputs/3
  FallTime/1 -> RSA_ADivB1/2

== system Renault_Library/Services ECU/Commonly_Used_Blocks/RSA_ServECU_EnaOutContact/SwitchWithoutSlope ==
  In1 : Inport
  Switch_Cmd : Inport  Port=2
  In0 : Inport  Port=3
  Enable : EnablePort  StatesWhenEnabling=reset
  Switch3 : Switch  Criteria=u2 ~= 0  RndMeth=Zero  InputSameDT=on
  Out1 : Outport  OutputWhenDisabled=reset  InitialOutput=0
  wires:
  In0/1 -> Switch3/3
  Switch3/1 -> Out1/1
  In1/1 -> Switch3/1
  Switch_Cmd/1 -> Switch3/2

== system Renault_Library/Services ECU/Commonly_Used_Blocks/RSA_ServECU_EnaOutContactSat/ActiveStateAdaptation ==
  LogicalState : Inport
  DataTypeConversion2 : DataTypeConversion  RndMeth=Zero
  LogicalOperator2 : Logic  Operator=NOT  OutDataTypeStr=boolean
  RiseTime3 : Constant  Value=LowActiveContact_C  OutDataTypeStr=Inherit: Inherit via back propagation  SampleTime=-1
  Switch1 : Switch  Criteria=u2 ~= 0  RndMeth=Zero  InputSameDT=on
  AdaptedLogicalState : Outport  OutputWhenDisabled=reset  InitialOutput=0
  wires:
  Switch1/1 -> AdaptedLogicalState/1
  LogicalState/1 -> DataTypeConversion2/1
  LogicalOperator2/1 -> Switch1/1
  RiseTime3/1 -> Switch1/2
  DataTypeConversion2/1 -> Switch1/3, LogicalOperator2/1

== system Renault_Library/Services ECU/Commonly_Used_Blocks/RSA_ServECU_EnaOutContactSat/SwitchWithSlope ==
  Switch_Cmd : Inport
  RiseTime : Inport  Port=2
  FallTime : Inport  Port=3
  LowLevelVoltage : Inport  Port=4
  HighLevelVoltage : Inport  Port=5
  Enable : EnablePort  StatesWhenEnabling=reset
  Add1 : Sum  Inputs=-+  RndMeth=Zero  InputSameDT=on
  Add2 : Sum  Inputs=-+  RndMeth=Zero  InputSameDT=on
  RSA_ADivB1 : Renault_Library/Complex blocks/Math Operations/RSA_ADivB  Tag=[LIB]
  RSA_ADivB2 : Renault_Library/Complex blocks/Math Operations/RSA_ADivB  Tag=[LIB]
  RSA_SlopeSwitch2Inputs : Renault_Library/Complex blocks/Signal Routing/RSA_SlopeSwitch2Inputs  Tag=[LIB]  st=SampleTimeSec_C
  Out1 : Outport  OutputWhenDisabled=reset  InitialOutput=0
  Out2 : Outport  Port=2  OutputWhenDisabled=reset  InitialOutput=0
  wires:
  LowLevelVoltage/1 -> [LowLevelVoltage], RSA_SlopeSwitch2Inputs/4
  HighLevelVoltage/1 -> [HighLevelVoltage], RSA_SlopeSwitch2Inputs/5
  Switch_Cmd/1 -> RSA_SlopeSwitch2Inputs/1
  RSA_SlopeSwitch2Inputs/1 -> Out1/1
  RSA_SlopeSwitch2Inputs/2 -> Out2/1
  [HighLevelVoltage] -> Add2/1
  Add2/1 -> RSA_ADivB1/1
  [LowLevelVoltage] -> Add2/2
  Add1/1 -> RSA_ADivB2/1
  [HighLevelVoltage] -> Add1/2
  [LowLevelVoltage] -> Add1/1
  RiseTime/1 -> RSA_ADivB2/2
  RSA_ADivB2/1 -> RSA_SlopeSwitch2Inputs/2
  RSA_ADivB1/1 -> RSA_SlopeSwitch2Inputs/3
  FallTime/1 -> RSA_ADivB1/2
